simulink model "benchmark_no_taylor"  (Simulink 8.8)
settings: Solver=ode45  StartTime=0.0  StopTime=1000  RelTol=1e-3  EnableMultiTasking=on
undefined symbols (not defined in the file or in its folder): sigbuilder_block
libraries: simulink x11, libturbines x10
(part 3 of 3)

== matlab function Wind Field/Wake7/Wake center and diameter ==
  data: uy input double; CT input double; p parameter double; w_c output double; w_d output double
function [w_c,w_d]=wake(uy,CT,p)
    % Todo: Change length(uy)*grid_size to farm size?
    %       Change such that parm is passed directly

    w_c=zeros(length(p.farm.x),1);
    w_d=zeros(length(p.farm.x),1);
    if CT < 1
        beta_w = (1+sqrt(1-CT))/(2*sqrt(1-CT));
    else
        beta_w = 1;
    end
    %beta_w = (1+sqrt(1-CT))/(2*sqrt(1-CT));
    %Find wake center and deficit for all other turbines
    for i=1:length(p.farm.x)
        delta_dist = p.farm.x(i)-p.wt.x;
        if(delta_dist>0) %Is turbine P downwind?
            w_d(i)= (beta_w^(p.k/2) +p.alpha*delta_dist/(2*p.wt.rotor.r))^(1/p.k)*2*p.wt.rotor.r;
            w_c(i)=wakecenter(uy,p.farm.x(i),p);%max(min(wakecenter(uy,p.farm.x(i),p),p.grid.ysize),1);
            
        else
            w_c(i)=p.wt.y;
            w_d(i)=0;
        end
    end
end


function wc=wakecenter(uy,px,p)
    
    %computer number of grid points to px
    it=ceil((px-p.wt.x)/p.grid.size);
    
    wc=p.wt.y; %wake center starts at turbine position
    
    for i=1:it %Compute the meandering for each grid point
        uysum=0;
        %compute the grid number for low meandering edge
        m_min=max(floor((wc-p.wt.meand.r(i))/p.grid.size)+1,1); 
        %compute the grid number for the high meandering edge
        m_max=min(ceil((wc+p.wt.meand.r(i))/p.grid.size)+1,length(uy)); 
        
        %average transversal velocity over the wake area
        for j=m_min:m_max
            uysum=uysum+uy(j);
        end
        uyavg=uysum/(m_max-m_min+1);
        
        % wake center filtered
        % Should be calculated from mean air speed vs uyavg and lowpass filtered
        % Redo filter computation
        % yeff=ts*uyavg=grid.size/uxmean*uyavg
        % wc=wc+alpha*uyavg
        
        %Uncomment to reproduce bug
        %wc=p.wt.y;
        
        %Uncomment to reproduce meandering limits
        %wc=min(max(wc,p.wt.y-150),p.wt.y+150);
        
        %Notice here ts such that wake moves with uyavg speed
        wc=wc+p.ts*uyavg;
    end
end

== matlab function Wind Field/Wind Speed7/Wind speed ==
  data: ux input double; def input double; p parameter double; uavg output double; upoint output double
function [uavg, upoint] = windspeed(ux,def,p) %#eml
% ux    is the wind vector at the x position of the turbine
% def   is the vector deficits at each turbine
% p     is a structure containing parameters  

uavg = def(p.wt.num)*sum(ux)/(p.wind_grid);
upoint = def(p.wt.num)*ux(ceil(p.wind_grid/2));

== matlab function Wind Field/Wake8/Wake center and diameter ==
  data: uy input double; CT input double; p parameter double; w_c output double; w_d output double
function [w_c,w_d]=wake(uy,CT,p)
    % Todo: Change length(uy)*grid_size to farm size?
    %       Change such that parm is passed directly

    w_c=zeros(length(p.farm.x),1);
    w_d=zeros(length(p.farm.x),1);
    if CT < 1
        beta_w = (1+sqrt(1-CT))/(2*sqrt(1-CT));
    else
        beta_w = 1;
    end
    %beta_w = (1+sqrt(1-CT))/(2*sqrt(1-CT));
    %Find wake center and deficit for all other turbines
    for i=1:length(p.farm.x)
        delta_dist = p.farm.x(i)-p.wt.x;
        if(delta_dist>0) %Is turbine P downwind?
            w_d(i)= (beta_w^(p.k/2) +p.alpha*delta_dist/(2*p.wt.rotor.r))^(1/p.k)*2*p.wt.rotor.r;
            w_c(i)=wakecenter(uy,p.farm.x(i),p);%max(min(wakecenter(uy,p.farm.x(i),p),p.grid.ysize),1);
            
        else
            w_c(i)=p.wt.y;
            w_d(i)=0;
        end
    end
end


function wc=wakecenter(uy,px,p)
    
    %computer number of grid points to px
    it=ceil((px-p.wt.x)/p.grid.size);
    
    wc=p.wt.y; %wake center starts at turbine position
    
    for i=1:it %Compute the meandering for each grid point
        uysum=0;
        %compute the grid number for low meandering edge
        m_min=max(floor((wc-p.wt.meand.r(i))/p.grid.size)+1,1); 
        %compute the grid number for the high meandering edge
        m_max=min(ceil((wc+p.wt.meand.r(i))/p.grid.size)+1,length(uy)); 
        
        %average transversal velocity over the wake area
        for j=m_min:m_max
            uysum=uysum+uy(j);
        end
        uyavg=uysum/(m_max-m_min+1);
        
        % wake center filtered
        % Should be calculated from mean air speed vs uyavg and lowpass filtered
        % Redo filter computation
        % yeff=ts*uyavg=grid.size/uxmean*uyavg
        % wc=wc+alpha*uyavg
        
        %Uncomment to reproduce bug
        %wc=p.wt.y;
        
        %Uncomment to reproduce meandering limits
        %wc=min(max(wc,p.wt.y-150),p.wt.y+150);
        
        %Notice here ts such that wake moves with uyavg speed
        wc=wc+p.ts*uyavg;
    end
end

== matlab function Wind Field/Wind Speed8/Wind speed ==
  data: ux input double; def input double; p parameter double; uavg output double; upoint output double
function [uavg, upoint] = windspeed(ux,def,p) %#eml
% ux    is the wind vector at the x position of the turbine
% def   is the vector deficits at each turbine
% p     is a structure containing parameters  

uavg = def(p.wt.num)*sum(ux)/(p.wind_grid);
upoint = def(p.wt.num)*ux(ceil(p.wind_grid/2));

== matlab function Wind Field/Wake9/Wake center and diameter ==
  data: uy input double; CT input double; p parameter double; w_c output double; w_d output double
function [w_c,w_d]=wake(uy,CT,p)
    % Todo: Change length(uy)*grid_size to farm size?
    %       Change such that parm is passed directly

    w_c=zeros(length(p.farm.x),1);
    w_d=zeros(length(p.farm.x),1);
    if CT < 1
        beta_w = (1+sqrt(1-CT))/(2*sqrt(1-CT));
    else
        beta_w = 1;
    end
    %beta_w = (1+sqrt(1-CT))/(2*sqrt(1-CT));
    %Find wake center and deficit for all other turbines
    for i=1:length(p.farm.x)
        delta_dist = p.farm.x(i)-p.wt.x;
        if(delta_dist>0) %Is turbine P downwind?
            w_d(i)= (beta_w^(p.k/2) +p.alpha*delta_dist/(2*p.wt.rotor.r))^(1/p.k)*2*p.wt.rotor.r;
            w_c(i)=wakecenter(uy,p.farm.x(i),p);%max(min(wakecenter(uy,p.farm.x(i),p),p.grid.ysize),1);
            
        else
            w_c(i)=p.wt.y;
            w_d(i)=0;
        end
    end
end


function wc=wakecenter(uy,px,p)
    
    %computer number of grid points to px
    it=ceil((px-p.wt.x)/p.grid.size);
    
    wc=p.wt.y; %wake center starts at turbine position
    
    for i=1:it %Compute the meandering for each grid point
        uysum=0;
        %compute the grid number for low meandering edge
        m_min=max(floor((wc-p.wt.meand.r(i))/p.grid.size)+1,1); 
        %compute the grid number for the high meandering edge
        m_max=min(ceil((wc+p.wt.meand.r(i))/p.grid.size)+1,length(uy)); 
        
        %average transversal velocity over the wake area
        for j=m_min:m_max
            uysum=uysum+uy(j);
        end
        uyavg=uysum/(m_max-m_min+1);
        
        % wake center filtered
        % Should be calculated from mean air speed vs uyavg and lowpass filtered
        % Redo filter computation
        % yeff=ts*uyavg=grid.size/uxmean*uyavg
        % wc=wc+alpha*uyavg
        
        %Uncomment to reproduce bug
        %wc=p.wt.y;
        
        %Uncomment to reproduce meandering limits
        %wc=min(max(wc,p.wt.y-150),p.wt.y+150);
        
        %Notice here ts such that wake moves with uyavg speed
        wc=wc+p.ts*uyavg;
    end
end

== matlab function Wind Field/Wind Speed9/Wind speed ==
  data: ux input double; def input double; p parameter double; uavg output double; upoint output double
function [uavg, upoint] = windspeed(ux,def,p) %#eml
% ux    is the wind vector at the x position of the turbine
% def   is the vector deficits at each turbine
% p     is a structure containing parameters  

uavg = def(p.wt.num)*sum(ux)/(p.wind_grid);
upoint = def(p.wt.num)*ux(ceil(p.wind_grid/2));

== matlab function Wind Field/Wake10/Wake center and diameter ==
  data: uy input double; CT input double; p parameter double; w_c output double; w_d output double
function [w_c,w_d]=wake(uy,CT,p)
    % Todo: Change length(uy)*grid_size to farm size?
    %       Change such that parm is passed directly

    w_c=zeros(length(p.farm.x),1);
    w_d=zeros(length(p.farm.x),1);
    if CT < 1
        beta_w = (1+sqrt(1-CT))/(2*sqrt(1-CT));
    else
        beta_w = 1;
    end
    %beta_w = (1+sqrt(1-CT))/(2*sqrt(1-CT));
    %Find wake center and deficit for all other turbines
    for i=1:length(p.farm.x)
        delta_dist = p.farm.x(i)-p.wt.x;
        if(delta_dist>0) %Is turbine P downwind?
            w_d(i)= (beta_w^(p.k/2) +p.alpha*delta_dist/(2*p.wt.rotor.r))^(1/p.k)*2*p.wt.rotor.r;
            w_c(i)=wakecenter(uy,p.farm.x(i),p);%max(min(wakecenter(uy,p.farm.x(i),p),p.grid.ysize),1);
            
        else
            w_c(i)=p.wt.y;
            w_d(i)=0;
        end
    end
end


function wc=wakecenter(uy,px,p)
    
    %computer number of grid points to px
    it=ceil((px-p.wt.x)/p.grid.size);
    
    wc=p.wt.y; %wake center starts at turbine position
    
    for i=1:it %Compute the meandering for each grid point
        uysum=0;
        %compute the grid number for low meandering edge
        m_min=max(floor((wc-p.wt.meand.r(i))/p.grid.size)+1,1); 
        %compute the grid number for the high meandering edge
        m_max=min(ceil((wc+p.wt.meand.r(i))/p.grid.size)+1,length(uy)); 
        
        %average transversal velocity over the wake area
        for j=m_min:m_max
            uysum=uysum+uy(j);
        end
        uyavg=uysum/(m_max-m_min+1);
        
        % wake center filtered
        % Should be calculated from mean air speed vs uyavg and lowpass filtered
        % Redo filter computation
        % yeff=ts*uyavg=grid.size/uxmean*uyavg
        % wc=wc+alpha*uyavg
        
        %Uncomment to reproduce bug
        %wc=p.wt.y;
        
        %Uncomment to reproduce meandering limits
        %wc=min(max(wc,p.wt.y-150),p.wt.y+150);
        
        %Notice here ts such that wake moves with uyavg speed
        wc=wc+p.ts*uyavg;
    end
end

== matlab function Wind Field/Wind Speed10/Wind speed ==
  data: ux input double; def input double; p parameter double; uavg output double; upoint output double
function [uavg, upoint] = windspeed(ux,def,p) %#eml
% ux    is the wind vector at the x position of the turbine
% def   is the vector deficits at each turbine
% p     is a structure containing parameters  

uavg = def(p.wt.num)*sum(ux)/(p.wind_grid);
upoint = def(p.wt.num)*ux(ceil(p.wind_grid/2));
